simulink model "CalcnOfCmptmtCtrlErr"  (R2022b)
settings: Solver=FixedStepDiscrete  StartTime=0.0  StopTime=10.0  FixedStep=1  RelTol=1e-3
settings Model: Creator=blue.ge  LastModifiedBy=blue.ge
settings DataIOCC: SaveFormat=StructureWithTime
settings OptimizationCC: DefaultParameterBehavior=Inlined  EnhancedBackFolding=on  PassReuseOutputArgsAs=Individual arguments  ZeroExternalMemoryAtStartup=off  ZeroInternalMemoryAtStartup=off  OptimizeBlockOrder=speed  DifferentSizesBufferReuse=on  OptimizationCustomize=off  EfficientTunableParamExpr=on
settings DebuggingCC: ParameterTunabilityLossMsg=error
settings ModelReferenceCC: ModelReferenceNumInstancesAllowed=Single
settings SFSimCC: MATLABDynamicMemAlloc=off
settings RTWCC: Description=AUTOSAR  SystemTargetFile=autosar.tlc  GenCodeOnly=on  TemplateMakefile=ert_default_tmf
settings CodeAppCC: ForceParamTrailComments=on  IgnoreCustomStorageClasses=off  OperatorAnnotations=on  SimulinkDataObjDesc=on  SFDataObjDesc=on  InsertBlockDesc=on
settings STFCustomTargetCC: TargetLangStandard=C99 (ISO)  MultiwordLength=256  ParMdlRefBuildCompliant=on  CompOptLevelCompliant=on  IncludeMdlTerminateFcn=off  CombineOutputUpdateFcns=on  SuppressErrorStatus=on  ERTCustomFileBanners=on  SupportNonFinite=off  SupportComplex=off  SupportContinuousTime=off  SupportNonInlinedSFcns=off  RemoveResetFunc=on  MATLABClassNameForMDSCustomization=Simulink.SoftwareTarget.ERTCustomization  AutosarCompliant=on  UseToolchainInfoCompliant=on  LookupTableObjectStructAxisOrder=2,1,3,4,...  LUTObjectStructOrderEvenSpacing=Size,Table,Breakpoints  SystemTargetFile=autosar.tlc
settings ERTTargetCC: TargetLangStandard=C99 (ISO)  ConcurrentExecutionCompliant=off  IncludeMdlTerminateFcn=off  SuppressErrorStatus=on  SupportNonFinite=off  SupportComplex=off  ModelStepFunctionPrototypeControlCompliant=off  CPPClassGenCompliant=off  AutosarCompliant=on  LookupTableObjectStructAxisOrder=2,1,3,4,...  LUTObjectStructOrderEvenSpacing=Size,Table,Breakpoints  InlinedParameterPlacement=NonHierarchical  ERTCustomFileTemplate=example_file_process.tlc

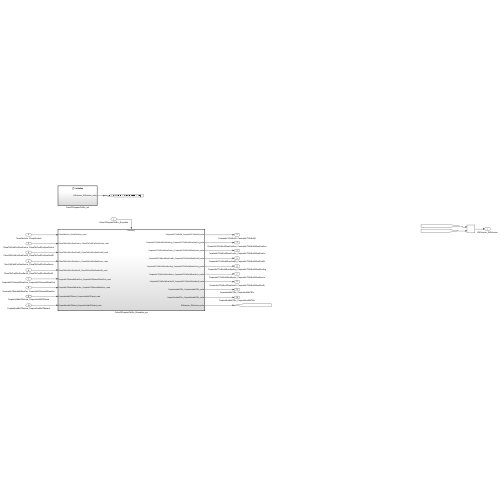
[thumbnail: Simulink's stored preview of the top-level diagram]
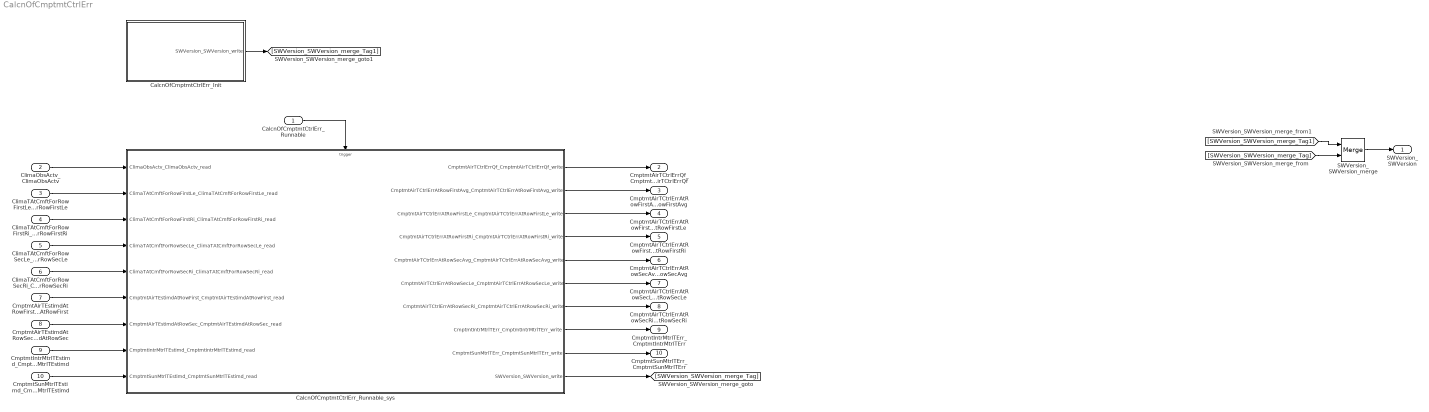
== system CalcnOfCmptmtCtrlErr ==
  CalcnOfCmptmtCtrlErr_Runnable : Inport  SampleTime=1  OutputFunctionCall=on
  ClimaObsActv_ClimaObsActv : Inport  Port=2  OutDataTypeStr=Boolean  PortDimensions=1
  ClimaTAtCmftForRowFirstLe_ClimaTAtCmftForRowFirstLe : Inport  Port=3  OutDataTypeStr=T30  PortDimensions=1
  ClimaTAtCmftForRowFirstRi_ClimaTAtCmftForRowFirstRi : Inport  Port=4  OutDataTypeStr=T30  PortDimensions=1
  ClimaTAtCmftForRowSecLe_ClimaTAtCmftForRowSecLe : Inport  Port=5  OutDataTypeStr=T30  PortDimensions=1
  ClimaTAtCmftForRowSecRi_ClimaTAtCmftForRowSecRi : Inport  Port=6  OutDataTypeStr=T30  PortDimensions=1
  CmptmtAirTEstimdAtRowFirst_CmptmtAirTEstimdAtRowFirst : Inport  Port=7  OutDataTypeStr=T30  PortDimensions=1
  CmptmtAirTEstimdAtRowSec_CmptmtAirTEstimdAtRowSec : Inport  Port=8  OutDataTypeStr=T30  PortDimensions=1
  CmptmtIntrMtrlTEstimd_CmptmtIntrMtrlTEstimd : Inport  Port=9  OutDataTypeStr=Bus: MtrlSnsrT  PortDimensions=1  BusOutputAsStruct=on
  CmptmtSunMtrlTEstimd_CmptmtSunMtrlTEstimd : Inport  Port=10  OutDataTypeStr=T30  PortDimensions=1
  CalcnOfCmptmtCtrlErr_Init : Subsystem
  CalcnOfCmptmtCtrlErr_Runnable_sys : Subsystem
  SWVersion_SWVersion_merge : Merge
  SWVersion_SWVersion : Outport  OutDataTypeStr=VCCUInt8  PortDimensions=11
  CmptmtAirTCtrlErrQf_CmptmtAirTCtrlErrQf : Outport  Port=2  OutDataTypeStr=Enum: HvacTIfQf  PortDimensions=1
  CmptmtAirTCtrlErrAtRowFirstAvg_CmptmtAirTCtrlErrAtRowFirstAvg : Outport  Port=3  OutDataTypeStr=T30  PortDimensions=1
  CmptmtAirTCtrlErrAtRowFirstLe_CmptmtAirTCtrlErrAtRowFirstLe : Outport  Port=4  OutDataTypeStr=T30  PortDimensions=1
  CmptmtAirTCtrlErrAtRowFirstRi_CmptmtAirTCtrlErrAtRowFirstRi : Outport  Port=5  OutDataTypeStr=T30  PortDimensions=1
  CmptmtAirTCtrlErrAtRowSecAvg_CmptmtAirTCtrlErrAtRowSecAvg : Outport  Port=6  OutDataTypeStr=T30  PortDimensions=1
  CmptmtAirTCtrlErrAtRowSecLe_CmptmtAirTCtrlErrAtRowSecLe : Outport  Port=7  OutDataTypeStr=T30  PortDimensions=1
  CmptmtAirTCtrlErrAtRowSecRi_CmptmtAirTCtrlErrAtRowSecRi : Outport  Port=8  OutDataTypeStr=T30  PortDimensions=1
  CmptmtIntrMtrlTErr_CmptmtIntrMtrlTErr : Outport  Port=9  OutDataTypeStr=T30  PortDimensions=1
  CmptmtSunMtrlTErr_CmptmtSunMtrlTErr : Outport  Port=10  OutDataTypeStr=T30  PortDimensions=1
  wires:
  ClimaObsActv_ClimaObsActv/1 -> CalcnOfCmptmtCtrlErr_Runnable_sys/1(ClimaObsActv_ClimaObsActv_read)
  ClimaTAtCmftForRowFirstLe_ClimaTAtCmftForRowFirstLe/1 -> CalcnOfCmptmtCtrlErr_Runnable_sys/2(ClimaTAtCmftForRowFirstLe_ClimaTAtCmftForRowFirstLe_read)
  ClimaTAtCmftForRowFirstRi_ClimaTAtCmftForRowFirstRi/1 -> CalcnOfCmptmtCtrlErr_Runnable_sys/3(ClimaTAtCmftForRowFirstRi_ClimaTAtCmftForRowFirstRi_read)
  ClimaTAtCmftForRowSecLe_ClimaTAtCmftForRowSecLe/1 -> CalcnOfCmptmtCtrlErr_Runnable_sys/4(ClimaTAtCmftForRowSecLe_ClimaTAtCmftForRowSecLe_read)
  ClimaTAtCmftForRowSecRi_ClimaTAtCmftForRowSecRi/1 -> CalcnOfCmptmtCtrlErr_Runnable_sys/5(ClimaTAtCmftForRowSecRi_ClimaTAtCmftForRowSecRi_read)
  CmptmtAirTEstimdAtRowFirst_CmptmtAirTEstimdAtRowFirst/1 -> CalcnOfCmptmtCtrlErr_Runnable_sys/6(CmptmtAirTEstimdAtRowFirst_CmptmtAirTEstimdAtRowFirst_read)
  CmptmtAirTEstimdAtRowSec_CmptmtAirTEstimdAtRowSec/1 -> CalcnOfCmptmtCtrlErr_Runnable_sys/7(CmptmtAirTEstimdAtRowSec_CmptmtAirTEstimdAtRowSec_read)
  CmptmtIntrMtrlTEstimd_CmptmtIntrMtrlTEstimd/1 -> CalcnOfCmptmtCtrlErr_Runnable_sys/8(CmptmtIntrMtrlTEstimd_CmptmtIntrMtrlTEstimd_read)
  CmptmtSunMtrlTEstimd_CmptmtSunMtrlTEstimd/1 -> CalcnOfCmptmtCtrlErr_Runnable_sys/9(CmptmtSunMtrlTEstimd_CmptmtSunMtrlTEstimd_read)
  CalcnOfCmptmtCtrlErr_Runnable_sys/1(CmptmtAirTCtrlErrQf_CmptmtAirTCtrlErrQf_write) -> CmptmtAirTCtrlErrQf_CmptmtAirTCtrlErrQf/1
  CalcnOfCmptmtCtrlErr_Runnable_sys/2(CmptmtAirTCtrlErrAtRowFirstAvg_CmptmtAirTCtrlErrAtRowFirstAvg_write) -> CmptmtAirTCtrlErrAtRowFirstAvg_CmptmtAirTCtrlErrAtRowFirstAvg/1
  CalcnOfCmptmtCtrlErr_Runnable_sys/3(CmptmtAirTCtrlErrAtRowFirstLe_CmptmtAirTCtrlErrAtRowFirstLe_write) -> CmptmtAirTCtrlErrAtRowFirstLe_CmptmtAirTCtrlErrAtRowFirstLe/1
  CalcnOfCmptmtCtrlErr_Runnable_sys/4(CmptmtAirTCtrlErrAtRowFirstRi_CmptmtAirTCtrlErrAtRowFirstRi_write) -> CmptmtAirTCtrlErrAtRowFirstRi_CmptmtAirTCtrlErrAtRowFirstRi/1
  CalcnOfCmptmtCtrlErr_Runnable_sys/5(CmptmtAirTCtrlErrAtRowSecAvg_CmptmtAirTCtrlErrAtRowSecAvg_write) -> CmptmtAirTCtrlErrAtRowSecAvg_CmptmtAirTCtrlErrAtRowSecAvg/1
  CalcnOfCmptmtCtrlErr_Runnable_sys/6(CmptmtAirTCtrlErrAtRowSecLe_CmptmtAirTCtrlErrAtRowSecLe_write) -> CmptmtAirTCtrlErrAtRowSecLe_CmptmtAirTCtrlErrAtRowSecLe/1
  CalcnOfCmptmtCtrlErr_Runnable_sys/7(CmptmtAirTCtrlErrAtRowSecRi_CmptmtAirTCtrlErrAtRowSecRi_write) -> CmptmtAirTCtrlErrAtRowSecRi_CmptmtAirTCtrlErrAtRowSecRi/1
  CalcnOfCmptmtCtrlErr_Runnable_sys/8(CmptmtIntrMtrlTErr_CmptmtIntrMtrlTErr_write) -> CmptmtIntrMtrlTErr_CmptmtIntrMtrlTErr/1
  CalcnOfCmptmtCtrlErr_Runnable_sys/9(CmptmtSunMtrlTErr_CmptmtSunMtrlTErr_write) -> CmptmtSunMtrlTErr_CmptmtSunMtrlTErr/1
  SWVersion_SWVersion_merge/1 -> SWVersion_SWVersion/1
  CalcnOfCmptmtCtrlErr_Runnable_sys/10(SWVersion_SWVersion_write) -> [SWVersion_SWVersion_merge_Tag]
  [SWVersion_SWVersion_merge_Tag] -> SWVersion_SWVersion_merge/2
  CalcnOfCmptmtCtrlErr_Init/1(SWVersion_SWVersion_write) -> [SWVersion_SWVersion_merge_Tag1]
  [SWVersion_SWVersion_merge_Tag1] -> SWVersion_SWVersion_merge/1
  CalcnOfCmptmtCtrlErr_Runnable/1 -> CalcnOfCmptmtCtrlErr_Runnable_sys/Trigger

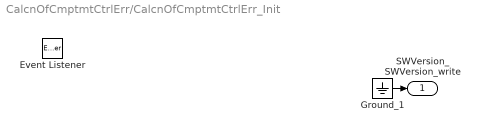
== system CalcnOfCmptmtCtrlErr/CalcnOfCmptmtCtrlErr_Init ==
  Event Listener : EventListener  EventName=reset
  Ground_1 : Ground
  SWVersion_SWVersion_write : Outport
  wires:
  Ground_1/1 -> SWVersion_SWVersion_write/1

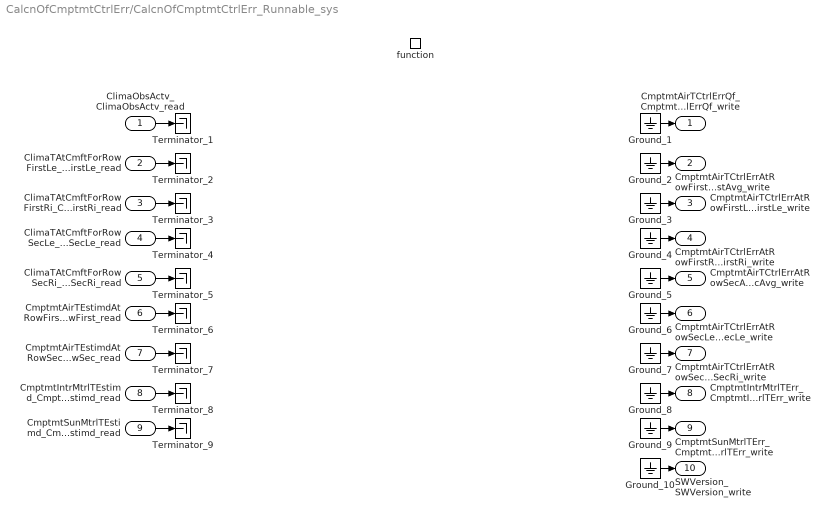
== system CalcnOfCmptmtCtrlErr/CalcnOfCmptmtCtrlErr_Runnable_sys ==
  ClimaObsActv_ClimaObsActv_read : Inport
  ClimaTAtCmftForRowFirstLe_ClimaTAtCmftForRowFirstLe_read : Inport  Port=2
  ClimaTAtCmftForRowFirstRi_ClimaTAtCmftForRowFirstRi_read : Inport  Port=3
  ClimaTAtCmftForRowSecLe_ClimaTAtCmftForRowSecLe_read : Inport  Port=4
  ClimaTAtCmftForRowSecRi_ClimaTAtCmftForRowSecRi_read : Inport  Port=5
  CmptmtAirTEstimdAtRowFirst_CmptmtAirTEstimdAtRowFirst_read : Inport  Port=6
  CmptmtAirTEstimdAtRowSec_CmptmtAirTEstimdAtRowSec_read : Inport  Port=7
  CmptmtIntrMtrlTEstimd_CmptmtIntrMtrlTEstimd_read : Inport  Port=8
  CmptmtSunMtrlTEstimd_CmptmtSunMtrlTEstimd_read : Inport  Port=9
  function : TriggerPort  TriggerType=function-call  VariantControl=(inherit)  SampleTimeType=periodic  SampleTime=-1
  Ground_{1..10} (10 alike) : Ground
  Terminator_{1..9} (9 alike) : Terminator
  CmptmtAirTCtrlErrQf_CmptmtAirTCtrlErrQf_write : Outport
      settings: EnsureOutportIsVirtual=on
  CmptmtAirTCtrlErrAtRowFirstAvg_CmptmtAirTCtrlErrAtRowFirstAvg_write : Outport  Port=2
      settings: EnsureOutportIsVirtual=on
  CmptmtAirTCtrlErrAtRowFirstLe_CmptmtAirTCtrlErrAtRowFirstLe_write : Outport  Port=3
      settings: EnsureOutportIsVirtual=on
  CmptmtAirTCtrlErrAtRowFirstRi_CmptmtAirTCtrlErrAtRowFirstRi_write : Outport  Port=4
      settings: EnsureOutportIsVirtual=on
  CmptmtAirTCtrlErrAtRowSecAvg_CmptmtAirTCtrlErrAtRowSecAvg_write : Outport  Port=5
      settings: EnsureOutportIsVirtual=on
  CmptmtAirTCtrlErrAtRowSecLe_CmptmtAirTCtrlErrAtRowSecLe_write : Outport  Port=6
      settings: EnsureOutportIsVirtual=on
  CmptmtAirTCtrlErrAtRowSecRi_CmptmtAirTCtrlErrAtRowSecRi_write : Outport  Port=7
      settings: EnsureOutportIsVirtual=on
  CmptmtIntrMtrlTErr_CmptmtIntrMtrlTErr_write : Outport  Port=8
      settings: EnsureOutportIsVirtual=on
  CmptmtSunMtrlTErr_CmptmtSunMtrlTErr_write : Outport  Port=9
      settings: EnsureOutportIsVirtual=on
  SWVersion_SWVersion_write : Outport  Port=10
      settings: EnsureOutportIsVirtual=on
  wires:
  ClimaObsActv_ClimaObsActv_read/1 -> Terminator_1/1
  ClimaTAtCmftForRowFirstLe_ClimaTAtCmftForRowFirstLe_read/1 -> Terminator_2/1
  ClimaTAtCmftForRowFirstRi_ClimaTAtCmftForRowFirstRi_read/1 -> Terminator_3/1
  ClimaTAtCmftForRowSecLe_ClimaTAtCmftForRowSecLe_read/1 -> Terminator_4/1
  ClimaTAtCmftForRowSecRi_ClimaTAtCmftForRowSecRi_read/1 -> Terminator_5/1
  CmptmtAirTEstimdAtRowFirst_CmptmtAirTEstimdAtRowFirst_read/1 -> Terminator_6/1
  CmptmtAirTEstimdAtRowSec_CmptmtAirTEstimdAtRowSec_read/1 -> Terminator_7/1
  CmptmtIntrMtrlTEstimd_CmptmtIntrMtrlTEstimd_read/1 -> Terminator_8/1
  CmptmtSunMtrlTEstimd_CmptmtSunMtrlTEstimd_read/1 -> Terminator_9/1
  Ground_1/1 -> CmptmtAirTCtrlErrQf_CmptmtAirTCtrlErrQf_write/1
  Ground_2/1 -> CmptmtAirTCtrlErrAtRowFirstAvg_CmptmtAirTCtrlErrAtRowFirstAvg_write/1
  Ground_3/1 -> CmptmtAirTCtrlErrAtRowFirstLe_CmptmtAirTCtrlErrAtRowFirstLe_write/1
  Ground_4/1 -> CmptmtAirTCtrlErrAtRowFirstRi_CmptmtAirTCtrlErrAtRowFirstRi_write/1
  Ground_5/1 -> CmptmtAirTCtrlErrAtRowSecAvg_CmptmtAirTCtrlErrAtRowSecAvg_write/1
  Ground_6/1 -> CmptmtAirTCtrlErrAtRowSecLe_CmptmtAirTCtrlErrAtRowSecLe_write/1
  Ground_7/1 -> CmptmtAirTCtrlErrAtRowSecRi_CmptmtAirTCtrlErrAtRowSecRi_write/1
  Ground_8/1 -> CmptmtIntrMtrlTErr_CmptmtIntrMtrlTErr_write/1
  Ground_9/1 -> CmptmtSunMtrlTErr_CmptmtSunMtrlTErr_write/1
  Ground_10/1 -> SWVersion_SWVersion_write/1
